simulink model "CCMVgaMotor"  (R2022b) (continued)
== system CCMVgaMotor == (continued)
  VentnActr12TrqSts_VentnActr12TrqSts : Inport  Port=351  OutDataTypeStr=Enum: VentnMotUrgcUnlck  PortDimensions=1
  VentnActr12UrgcExist_VentnActr12UrgcExist : Inport  Port=352  OutDataTypeStr=Enum: VentnActr01UrgcExist  PortDimensions=1
  VentnActr12UrgcPson_VentnActr12UrgcPson : Inport  Port=353  OutDataTypeStr=Enum: VentnMotUrgcPson  PortDimensions=1
  VentnActr12UrgcSts_VentnActr12UrgcSts : Inport  Port=354  OutDataTypeStr=Enum: VentnMotUrgcUnlck  PortDimensions=1
  VentnActr12VoltErr_VentnActr12VoltErr : Inport  Port=355  OutDataTypeStr=Enum: VentnActr01VoltErr  PortDimensions=1
  VentnActr13ActPson_VentnActr13ActPson : Inport  Port=356  OutDataTypeStr=UInt16  PortDimensions=1
  VentnActr13ActPson_VentnActr13ActPson_IsUpdated : Inport  Port=357  OutDataTypeStr=boolean  PortDimensions=1
  VentnActr13BlckInd_VentnActr13BlckInd : Inport  Port=358  OutDataTypeStr=Enum: VentnActr01BlckInd  PortDimensions=1
  VentnActr13BlckSts_VentnActr13BlckSts : Inport  Port=359  OutDataTypeStr=Enum: VentnActr01BlckSts  PortDimensions=1
  VentnActr13BusAdr_VentnActr13BusAdr : Inport  Port=360  OutDataTypeStr=UInt8  PortDimensions=1
  VentnActr13CoilSts_VentnActr13CoilSts : Inport  Port=361  OutDataTypeStr=Enum: VentnMotUrgcUnlck  PortDimensions=1
  VentnActr13DrvDir_VentnActr13DrvDir : Inport  Port=362  OutDataTypeStr=Enum: VentnActr01DrvDir  PortDimensions=1
  VentnActr13ElecErr_VentnActr13ElecErr : Inport  Port=363  OutDataTypeStr=Enum: VentnActr01ElecErr  PortDimensions=1
  VentnActr13Mode_VentnActr13Mode : Inport  Port=364  OutDataTypeStr=Enum: VentnMotMode  PortDimensions=1
  VentnActr13MovDir_VentnActr13MovDir : Inport  Port=365  OutDataTypeStr=Enum: VentnMotDir  PortDimensions=1
  VentnActr13OvrTempErr_VentnActr13OvrTempErr : Inport  Port=366  OutDataTypeStr=Enum: VentnActr01OvrTempErr  PortDimensions=1
  VentnActr13PsonSts_VentnActr13PsonSts : Inport  Port=367  OutDataTypeStr=Enum: VentnMotPsonAvl  PortDimensions=1
  VentnActr13ResetSts_VentnActr13ResetSts : Inport  Port=368  OutDataTypeStr=Enum: VentnActr01ResetSts  PortDimensions=1
  VentnActr13SpclFct_VentnActr13SpclFct : Inport  Port=369  OutDataTypeStr=Enum: VentnActr01SpclFct  PortDimensions=1
  VentnActr13SpdSts_VentnActr13SpdSts : Inport  Port=370  OutDataTypeStr=Enum: VentnActr01SpdSts  PortDimensions=1
  VentnActr13TrqSts_VentnActr13TrqSts : Inport  Port=371  OutDataTypeStr=Enum: VentnMotUrgcUnlck  PortDimensions=1
  VentnActr13UrgcExist_VentnActr13UrgcExist : Inport  Port=372  OutDataTypeStr=Enum: VentnActr01UrgcExist  PortDimensions=1
  VentnActr13UrgcPson_VentnActr13UrgcPson : Inport  Port=373  OutDataTypeStr=Enum: VentnMotUrgcPson  PortDimensions=1
  VentnActr13UrgcSts_VentnActr13UrgcSts : Inport  Port=374  OutDataTypeStr=Enum: VentnMotUrgcUnlck  PortDimensions=1
  VentnActr13VoltErr_VentnActr13VoltErr : Inport  Port=375  OutDataTypeStr=Enum: VentnActr01VoltErr  PortDimensions=1
  VentnActr14ActPson_VentnActr14ActPson : Inport  Port=376  OutDataTypeStr=UInt16  PortDimensions=1
  VentnActr14ActPson_VentnActr14ActPson_IsUpdated : Inport  Port=377  OutDataTypeStr=boolean  PortDimensions=1
  VentnActr14BlckInd_VentnActr14BlckInd : Inport  Port=378  OutDataTypeStr=Enum: VentnActr01BlckInd  PortDimensions=1
  VentnActr14BlckSts_VentnActr14BlckSts : Inport  Port=379  OutDataTypeStr=Enum: VentnActr01BlckSts  PortDimensions=1
  VentnActr14BusAdr_VentnActr14BusAdr : Inport  Port=380  OutDataTypeStr=UInt8  PortDimensions=1
  VentnActr14CoilSts_VentnActr14CoilSts : Inport  Port=381  OutDataTypeStr=Enum: VentnMotUrgcUnlck  PortDimensions=1
  VentnActr14DrvDir_VentnActr14DrvDir : Inport  Port=382  OutDataTypeStr=Enum: VentnActr01DrvDir  PortDimensions=1
  VentnActr14ElecErr_VentnActr14ElecErr : Inport  Port=383  OutDataTypeStr=Enum: VentnActr01ElecErr  PortDimensions=1
  VentnActr14Mode_VentnActr14Mode : Inport  Port=384  OutDataTypeStr=Enum: VentnMotMode  PortDimensions=1
  VentnActr14MovDir_VentnActr14MovDir : Inport  Port=385  OutDataTypeStr=Enum: VentnMotDir  PortDimensions=1
  VentnActr14OvrTempErr_VentnActr14OvrTempErr : Inport  Port=386  OutDataTypeStr=Enum: VentnActr01OvrTempErr  PortDimensions=1
  VentnActr14PsonSts_VentnActr14PsonSts : Inport  Port=387  OutDataTypeStr=Enum: VentnMotPsonAvl  PortDimensions=1
  VentnActr14ResetSts_VentnActr14ResetSts : Inport  Port=388  OutDataTypeStr=Enum: VentnActr01ResetSts  PortDimensions=1
  VentnActr14SpclFct_VentnActr14SpclFct : Inport  Port=389  OutDataTypeStr=Enum: VentnActr01SpclFct  PortDimensions=1
  VentnActr14SpdSts_VentnActr14SpdSts : Inport  Port=390  OutDataTypeStr=Enum: VentnActr01SpdSts  PortDimensions=1
  VentnActr14TrqSts_VentnActr14TrqSts : Inport  Port=391  OutDataTypeStr=Enum: VentnMotUrgcUnlck  PortDimensions=1
  VentnActr14UrgcExist_VentnActr14UrgcExist : Inport  Port=392  OutDataTypeStr=Enum: VentnActr01UrgcExist  PortDimensions=1
  VentnActr14UrgcPson_VentnActr14UrgcPson : Inport  Port=393  OutDataTypeStr=Enum: VentnMotUrgcPson  PortDimensions=1
  VentnActr14UrgcSts_VentnActr14UrgcSts : Inport  Port=394  OutDataTypeStr=Enum: VentnMotUrgcUnlck  PortDimensions=1
  VentnActr14VoltErr_VentnActr14VoltErr : Inport  Port=395  OutDataTypeStr=Enum: VentnActr01VoltErr  PortDimensions=1
  VentnActr15ActPson_VentnActr15ActPson : Inport  Port=396  OutDataTypeStr=UInt16  PortDimensions=1
  VentnActr15ActPson_VentnActr15ActPson_IsUpdated : Inport  Port=397  OutDataTypeStr=boolean  PortDimensions=1
  VentnActr15BlckInd_VentnActr15BlckInd : Inport  Port=398  OutDataTypeStr=Enum: VentnActr01BlckInd  PortDimensions=1
  VentnActr15BlckSts_VentnActr15BlckSts : Inport  Port=399  OutDataTypeStr=Enum: VentnActr01BlckSts  PortDimensions=1
  VentnActr15BusAdr_VentnActr15BusAdr : Inport  Port=400  OutDataTypeStr=UInt8  PortDimensions=1
  VentnActr15CoilSts_VentnActr15CoilSts : Inport  Port=401  OutDataTypeStr=Enum: VentnMotUrgcUnlck  PortDimensions=1
  VentnActr15DrvDir_VentnActr15DrvDir : Inport  Port=402  OutDataTypeStr=Enum: VentnActr01DrvDir  PortDimensions=1
  VentnActr15ElecErr_VentnActr15ElecErr : Inport  Port=403  OutDataTypeStr=Enum: VentnActr01ElecErr  PortDimensions=1
  VentnActr15Mode_VentnActr15Mode : Inport  Port=404  OutDataTypeStr=Enum: VentnMotMode  PortDimensions=1
  VentnActr15MovDir_VentnActr15MovDir : Inport  Port=405  OutDataTypeStr=Enum: VentnMotDir  PortDimensions=1
  VentnActr15OvrTempErr_VentnActr15OvrTempErr : Inport  Port=406  OutDataTypeStr=Enum: VentnActr01OvrTempErr  PortDimensions=1
  VentnActr15PsonSts_VentnActr15PsonSts : Inport  Port=407  OutDataTypeStr=Enum: VentnMotPsonAvl  PortDimensions=1
  VentnActr15ResetSts_VentnActr15ResetSts : Inport  Port=408  OutDataTypeStr=Enum: VentnActr01ResetSts  PortDimensions=1
  VentnActr15SpclFct_VentnActr15SpclFct : Inport  Port=409  OutDataTypeStr=Enum: VentnActr01SpclFct  PortDimensions=1
  VentnActr15SpdSts_VentnActr15SpdSts : Inport  Port=410  OutDataTypeStr=Enum: VentnActr01SpdSts  PortDimensions=1
  VentnActr15TrqSts_VentnActr15TrqSts : Inport  Port=411  OutDataTypeStr=Enum: VentnMotUrgcUnlck  PortDimensions=1
  VentnActr15UrgcExist_VentnActr15UrgcExist : Inport  Port=412  OutDataTypeStr=Enum: VentnActr01UrgcExist  PortDimensions=1
  VentnActr15UrgcPson_VentnActr15UrgcPson : Inport  Port=413  OutDataTypeStr=Enum: VentnMotUrgcPson  PortDimensions=1
  VentnActr15UrgcSts_VentnActr15UrgcSts : Inport  Port=414  OutDataTypeStr=Enum: VentnMotUrgcUnlck  PortDimensions=1
  VentnActr15VoltErr_VentnActr15VoltErr : Inport  Port=415  OutDataTypeStr=Enum: VentnActr01VoltErr  PortDimensions=1
  VentnActr16ActPson_VentnActr16ActPson : Inport  Port=416  OutDataTypeStr=UInt16  PortDimensions=1
  VentnActr16BlckInd_VentnActr16BlckInd : Inport  Port=417  OutDataTypeStr=Enum: VentnActr01BlckInd  PortDimensions=1
  VentnActr16BlckSts_VentnActr16BlckSts : Inport  Port=418  OutDataTypeStr=Enum: VentnActr01BlckSts  PortDimensions=1
  VentnActr16BusAdr_VentnActr16BusAdr : Inport  Port=419  OutDataTypeStr=UInt8  PortDimensions=1
  VentnActr16CoilSts_VentnActr16CoilSts : Inport  Port=420  OutDataTypeStr=Enum: VentnMotUrgcUnlck  PortDimensions=1
  VentnActr16DrvDir_VentnActr16DrvDir : Inport  Port=421  OutDataTypeStr=Enum: VentnActr01DrvDir  PortDimensions=1
  VentnActr16ElecErr_VentnActr16ElecErr : Inport  Port=422  OutDataTypeStr=Enum: VentnActr01ElecErr  PortDimensions=1
  VentnActr16Mode_VentnActr16Mode : Inport  Port=423  OutDataTypeStr=Enum: VentnMotMode  PortDimensions=1
  VentnActr16MovDir_VentnActr16MovDir : Inport  Port=424  OutDataTypeStr=Enum: VentnMotDir  PortDimensions=1
  VentnActr16OvrTempErr_VentnActr16OvrTempErr : Inport  Port=425  OutDataTypeStr=Enum: VentnActr01OvrTempErr  PortDimensions=1
  VentnActr16PsonSts_VentnActr16PsonSts : Inport  Port=426  OutDataTypeStr=Enum: VentnMotPsonAvl  PortDimensions=1
  VentnActr16ResetSts_VentnActr16ResetSts : Inport  Port=427  OutDataTypeStr=Enum: VentnActr01ResetSts  PortDimensions=1
  VentnActr16SpclFct_VentnActr16SpclFct : Inport  Port=428  OutDataTypeStr=Enum: VentnActr01SpclFct  PortDimensions=1
  VentnActr16SpdSts_VentnActr16SpdSts : Inport  Port=429  OutDataTypeStr=Enum: VentnActr01SpdSts  PortDimensions=1
  VentnActr16TrqSts_VentnActr16TrqSts : Inport  Port=430  OutDataTypeStr=Enum: VentnMotUrgcUnlck  PortDimensions=1
  VentnActr16UrgcExist_VentnActr16UrgcExist : Inport  Port=431  OutDataTypeStr=Enum: VentnActr01UrgcExist  PortDimensions=1
  VentnActr16UrgcPson_VentnActr16UrgcPson : Inport  Port=432  OutDataTypeStr=Enum: VentnMotUrgcPson  PortDimensions=1
  VentnActr16UrgcSts_VentnActr16UrgcSts : Inport  Port=433  OutDataTypeStr=Enum: VentnMotUrgcUnlck  PortDimensions=1
  VentnActr16VoltErr_VentnActr16VoltErr : Inport  Port=434  OutDataTypeStr=Enum: VentnActr01VoltErr  PortDimensions=1
  VentnActr17ActPson_VentnActr17ActPson : Inport  Port=435  OutDataTypeStr=UInt16  PortDimensions=1
  VentnActr17ActPson_VentnActr17ActPson_IsUpdated : Inport  Port=436  OutDataTypeStr=boolean  PortDimensions=1
  VentnActr17BlckInd_VentnActr17BlckInd : Inport  Port=437  OutDataTypeStr=Enum: VentnActr01BlckInd  PortDimensions=1
  VentnActr17BlckSts_VentnActr17BlckSts : Inport  Port=438  OutDataTypeStr=Enum: VentnActr01BlckSts  PortDimensions=1
  VentnActr17BusAdr_VentnActr17BusAdr : Inport  Port=439  OutDataTypeStr=UInt8  PortDimensions=1
  VentnActr17CoilSts_VentnActr17CoilSts : Inport  Port=440  OutDataTypeStr=Enum: VentnMotUrgcUnlck  PortDimensions=1
  VentnActr17DrvDir_VentnActr17DrvDir : Inport  Port=441  OutDataTypeStr=Enum: VentnActr01DrvDir  PortDimensions=1
  VentnActr17ElecErr_VentnActr17ElecErr : Inport  Port=442  OutDataTypeStr=Enum: VentnActr01ElecErr  PortDimensions=1
  VentnActr17Mode_VentnActr17Mode : Inport  Port=443  OutDataTypeStr=Enum: VentnMotMode  PortDimensions=1
  VentnActr17MovDir_VentnActr17MovDir : Inport  Port=444  OutDataTypeStr=Enum: VentnMotDir  PortDimensions=1
  VentnActr17OvrTempErr_VentnActr17OvrTempErr : Inport  Port=445  OutDataTypeStr=Enum: VentnActr01OvrTempErr  PortDimensions=1
  VentnActr17PsonSts_VentnActr17PsonSts : Inport  Port=446  OutDataTypeStr=Enum: VentnMotPsonAvl  PortDimensions=1
  VentnActr17ResetSts_VentnActr17ResetSts : Inport  Port=447  OutDataTypeStr=Enum: VentnActr01ResetSts  PortDimensions=1
  VentnActr17SpclFct_VentnActr17SpclFct : Inport  Port=448  OutDataTypeStr=Enum: VentnActr01SpclFct  PortDimensions=1
  VentnActr17SpdSts_VentnActr17SpdSts : Inport  Port=449  OutDataTypeStr=Enum: VentnActr01SpdSts  PortDimensions=1
  VentnActr17TrqSts_VentnActr17TrqSts : Inport  Port=450  OutDataTypeStr=Enum: VentnMotUrgcUnlck  PortDimensions=1
  VentnActr17UrgcExist_VentnActr17UrgcExist : Inport  Port=451  OutDataTypeStr=Enum: VentnActr01UrgcExist  PortDimensions=1
  VentnActr17UrgcPson_VentnActr17UrgcPson : Inport  Port=452  OutDataTypeStr=Enum: VentnMotUrgcPson  PortDimensions=1
  VentnActr17UrgcSts_VentnActr17UrgcSts : Inport  Port=453  OutDataTypeStr=Enum: VentnMotUrgcUnlck  PortDimensions=1
  VentnActr17VoltErr_VentnActr17VoltErr : Inport  Port=454  OutDataTypeStr=Enum: VentnActr01VoltErr  PortDimensions=1
  VentnActr18ActPson_VentnActr18ActPson : Inport  Port=455  OutDataTypeStr=UInt16  PortDimensions=1
  VentnActr18BlckInd_VentnActr18BlckInd : Inport  Port=456  OutDataTypeStr=Enum: VentnActr01BlckInd  PortDimensions=1
  VentnActr18BlckSts_VentnActr18BlckSts : Inport  Port=457  OutDataTypeStr=Enum: VentnActr01BlckInd  PortDimensions=1
  VentnActr18BusAdr_VentnActr18BusAdr : Inport  Port=458  OutDataTypeStr=UInt8  PortDimensions=1
  VentnActr18CoilSts_VentnActr18CoilSts : Inport  Port=459  OutDataTypeStr=Enum: VentnMotUrgcUnlck  PortDimensions=1
  VentnActr18DrvDir_VentnActr18DrvDir : Inport  Port=460  OutDataTypeStr=Enum: VentnActr01DrvDir  PortDimensions=1
  VentnActr18ElecErr_VentnActr18ElecErr : Inport  Port=461  OutDataTypeStr=Enum: VentnActr01ElecErr  PortDimensions=1
  VentnActr18Mode_VentnActr18Mode : Inport  Port=462  OutDataTypeStr=Enum: VentnMotMode  PortDimensions=1
  VentnActr18MovDir_VentnActr18MovDir : Inport  Port=463  OutDataTypeStr=Enum: VentnMotDir  PortDimensions=1
  VentnActr18OvrTempErr_VentnActr18OvrTempErr : Inport  Port=464  OutDataTypeStr=Enum: VentnActr01OvrTempErr  PortDimensions=1
  VentnActr18PsonSts_VentnActr18PsonSts : Inport  Port=465  OutDataTypeStr=Enum: VentnMotPsonAvl  PortDimensions=1
  VentnActr18ResetSts_VentnActr18ResetSts : Inport  Port=466  OutDataTypeStr=Enum: VentnActr01ResetSts  PortDimensions=1
  VentnActr18SpclFct_VentnActr18SpclFct : Inport  Port=467  OutDataTypeStr=Enum: VentnActr01SpclFct  PortDimensions=1
  VentnActr18SpdSts_VentnActr18SpdSts : Inport  Port=468  OutDataTypeStr=Enum: VentnActr01SpdSts  PortDimensions=1
  VentnActr18TrqSts_VentnActr18TrqSts : Inport  Port=469  OutDataTypeStr=Enum: VentnMotUrgcUnlck  PortDimensions=1
  VentnActr18UrgcExist_VentnActr18UrgcExist : Inport  Port=470  OutDataTypeStr=Enum: VentnActr01UrgcExist  PortDimensions=1
  VentnActr18UrgcPson_VentnActr18UrgcPson : Inport  Port=471  OutDataTypeStr=Enum: VentnMotUrgcPson  PortDimensions=1
  VentnActr18UrgcSts_VentnActr18UrgcSts : Inport  Port=472  OutDataTypeStr=Enum: VentnMotUrgcUnlck  PortDimensions=1
  VentnActr18VoltErr_VentnActr18VoltErr : Inport  Port=473  OutDataTypeStr=Enum: VentnActr01ElecErr  PortDimensions=1
  VentnActr19ActPson_VentnActr19ActPson : Inport  Port=474  OutDataTypeStr=UInt16  PortDimensions=1
  VentnActr19ActPson_VentnActr19ActPson_IsUpdated : Inport  Port=475  OutDataTypeStr=boolean  PortDimensions=1
  VentnActr19BlckInd_VentnActr19BlckInd : Inport  Port=476  OutDataTypeStr=Enum: VentnActr01BlckInd  PortDimensions=1
  VentnActr19BlckSts_VentnActr19BlckSts : Inport  Port=477  OutDataTypeStr=Enum: VentnActr01BlckInd  PortDimensions=1
  VentnActr19BusAdr_VentnActr19BusAdr : Inport  Port=478  OutDataTypeStr=UInt8  PortDimensions=1
  VentnActr19CoilSts_VentnActr19CoilSts : Inport  Port=479  OutDataTypeStr=Enum: VentnMotUrgcUnlck  PortDimensions=1
  VentnActr19DrvDir_VentnActr19DrvDir : Inport  Port=480  OutDataTypeStr=Enum: VentnActr01DrvDir  PortDimensions=1
  VentnActr19ElecErr_VentnActr19ElecErr : Inport  Port=481  OutDataTypeStr=Enum: VentnActr01ElecErr  PortDimensions=1
  VentnActr19Mode_VentnActr19Mode : Inport  Port=482  OutDataTypeStr=Enum: VentnMotMode  PortDimensions=1
  VentnActr19MovDir_VentnActr19MovDir : Inport  Port=483  OutDataTypeStr=Enum: VentnMotDir  PortDimensions=1
  VentnActr19OvrTempErr_VentnActr19OvrTempErr : Inport  Port=484  OutDataTypeStr=Enum: VentnActr01OvrTempErr  PortDimensions=1
  VentnActr19PsonSts_VentnActr19PsonSts : Inport  Port=485  OutDataTypeStr=Enum: VentnMotPsonAvl  PortDimensions=1
  VentnActr19ResetSts_VentnActr19ResetSts : Inport  Port=486  OutDataTypeStr=Enum: VentnActr01ResetSts  PortDimensions=1
  VentnActr19SpclFct_VentnActr19SpclFct : Inport  Port=487  OutDataTypeStr=Enum: VentnActr01SpclFct  PortDimensions=1
  VentnActr19SpdSts_VentnActr19SpdSts : Inport  Port=488  OutDataTypeStr=Enum: VentnActr01SpdSts  PortDimensions=1
  VentnActr19TrqSts_VentnActr19TrqSts : Inport  Port=489  OutDataTypeStr=Enum: VentnMotUrgcUnlck  PortDimensions=1
  VentnActr19UrgcExist_VentnActr19UrgcExist : Inport  Port=490  OutDataTypeStr=Enum: VentnActr01UrgcExist  PortDimensions=1
  VentnActr19UrgcPson_VentnActr19UrgcPson : Inport  Port=491  OutDataTypeStr=Enum: VentnMotUrgcPson  PortDimensions=1
  VentnActr19UrgcSts_VentnActr19UrgcSts : Inport  Port=492  OutDataTypeStr=Enum: VentnMotUrgcUnlck  PortDimensions=1
  VentnActr19VoltErr_VentnActr19VoltErr : Inport  Port=493  OutDataTypeStr=Enum: VentnActr01VoltErr  PortDimensions=1
  VentnActr20ActPson_VentnActr20ActPson : Inport  Port=494  OutDataTypeStr=UInt16  PortDimensions=1
  VentnActr20ActPson_VentnActr20ActPson_IsUpdated : Inport  Port=495  OutDataTypeStr=boolean  PortDimensions=1
  VentnActr20BlckInd_VentnActr20BlckInd : Inport  Port=496  OutDataTypeStr=Enum: VentnActr01BlckInd  PortDimensions=1
  VentnActr20BlckSts_VentnActr20BlckSts : Inport  Port=497  OutDataTypeStr=Enum: VentnActr01BlckSts  PortDimensions=1
  VentnActr20BusAdr_VentnActr20BusAdr : Inport  Port=498  OutDataTypeStr=UInt8  PortDimensions=1
  VentnActr20CoilSts_VentnActr20CoilSts : Inport  Port=499  OutDataTypeStr=Enum: VentnMotUrgcUnlck  PortDimensions=1
  VentnActr20DrvDir_VentnActr20DrvDir : Inport  Port=500  OutDataTypeStr=Enum: VentnActr01DrvDir  PortDimensions=1
  VentnActr20ElecErr_VentnActr20ElecErr : Inport  Port=501  OutDataTypeStr=Enum: VentnActr01ElecErr  PortDimensions=1
  VentnActr20Mode_VentnActr20Mode : Inport  Port=502  OutDataTypeStr=Enum: VentnMotMode  PortDimensions=1
  VentnActr20MovDir_VentnActr20MovDir : Inport  Port=503  OutDataTypeStr=Enum: VentnMotDir  PortDimensions=1
  VentnActr20OvrTempErr_VentnActr20OvrTempErr : Inport  Port=504  OutDataTypeStr=Enum: VentnActr01OvrTempErr  PortDimensions=1
  VentnActr20PsonSts_VentnActr20PsonSts : Inport  Port=505  OutDataTypeStr=Enum: VentnMotPsonAvl  PortDimensions=1
  VentnActr20ResetSts_VentnActr20ResetSts : Inport  Port=506  OutDataTypeStr=Enum: VentnActr01ResetSts  PortDimensions=1
  VentnActr20SpclFct_VentnActr20SpclFct : Inport  Port=507  OutDataTypeStr=Enum: VentnActr01SpclFct  PortDimensions=1
  VentnActr20SpdSts_VentnActr20SpdSts : Inport  Port=508  OutDataTypeStr=Enum: VentnActr01SpdSts  PortDimensions=1
  VentnActr20TrqSts_VentnActr20TrqSts : Inport  Port=509  OutDataTypeStr=Enum: VentnMotUrgcUnlck  PortDimensions=1
  VentnActr20UrgcExist_VentnActr20UrgcExist : Inport  Port=510  OutDataTypeStr=Enum: VentnActr01UrgcExist  PortDimensions=1
  VentnActr20UrgcPson_VentnActr20UrgcPson : Inport  Port=511  OutDataTypeStr=Enum: VentnMotUrgcPson  PortDimensions=1
  VentnActr20UrgcSts_VentnActr20UrgcSts : Inport  Port=512  OutDataTypeStr=Enum: VentnMotUrgcUnlck  PortDimensions=1
  VentnActr20VoltErr_VentnActr20VoltErr : Inport  Port=513  OutDataTypeStr=Enum: VentnActr01VoltErr  PortDimensions=1
  VGAStopDuePowerLimit_VGAStopDuePowerLimit : Inport  Port=514  OutDataTypeStr=Boolean  PortDimensions=1
  BswLifeCycle_LifeCycle : Inport  Port=515  OutDataTypeStr=Enum: CCMLifeCycleModeGroup  PortDimensions=1
  CCMVGAMotor_VentnMotAdr2_sys : Subsystem
  CCMVGAMotor_VentnMotAdr_sys : Subsystem
  CCMVgaMotor_50msRunnable_sys : Subsystem
  CCMVgaMotor_Init : Subsystem
  CCMVgaMotor_Runnable_sys : Subsystem
  NvMBlockVgaMtrCalData : DataStoreMemory  DataStoreName=NvMBlockVgaMtrCalData  InitialValue=NvMBlockVgaMtrCalDataInit  ShowAdditionalParam=off  Dimensions=210  StateMustResolveToSignalObject=on
  VgaCaliServive : Subsystem
  VgaDtcService : Subsystem
  VgaIOCtrlService : Subsystem
  VgaNvmService : Subsystem
  VgaParaService : Subsystem
  VentnMotAdr_VentnMotAdr : Outport  OutDataTypeStr=UInt8  PortDimensions=1
  VentnMotAdr2_VentnMotAdr2 : Outport  Port=2  OutDataTypeStr=UInt8  PortDimensions=1
  VentnMotBlockCmd_VentnMotBlockCmd : Outport  Port=3  OutDataTypeStr=Enum: VentnMotBlockCmd  PortDimensions=1
  VentnMotBlockCmd2_VentnMotBlockCmd2 : Outport  Port=4  OutDataTypeStr=Enum: VentnMotBlockCmd  PortDimensions=1
  VentnMotClearReq_VentnMotClearReq : Outport  Port=5  OutMin=0  OutMax=15  OutDataTypeStr=UInt4  PortDimensions=1
  VentnMotClearReq2_VentnMotClearReq2 : Outport  Port=6  OutMin=0  OutMax=15  OutDataTypeStr=UInt4  PortDimensions=1
  VentnMotCoilPwrOn_VentnMotCoilPwrOn : Outport  Port=7  OutDataTypeStr=Enum: VentnMotUrgcUnlck  PortDimensions=1
  VentnMotCoilPwrOn2_VentnMotCoilPwrOn2 : Outport  Port=8  OutDataTypeStr=Enum: VentnMotUrgcUnlck  PortDimensions=1
  VentnMotDir_VentnMotDir : Outport  Port=9  OutDataTypeStr=Enum: VentnMotDir  PortDimensions=1
  VentnMotDir2_VentnMotDir2 : Outport  Port=10  OutDataTypeStr=Enum: VentnMotDir  PortDimensions=1
  VentnMotMode_VentnMotMode : Outport  Port=11  OutDataTypeStr=Enum: VentnMotMode  PortDimensions=1
  VentnMotMode2_VentnMotMode2 : Outport  Port=12  OutDataTypeStr=Enum: VentnMotMode  PortDimensions=1
  VentnMotPsonAvl_VentnMotPsonAvl : Outport  Port=13  OutDataTypeStr=Enum: VentnMotPsonAvl  PortDimensions=1
  VentnMotPsonAvl2_VentnMotPsonAvl2 : Outport  Port=14  OutDataTypeStr=Enum: VentnMotPsonAvl  PortDimensions=1
  VentnMotSaveData_VentnMotSaveData : Outport  Port=15  OutDataTypeStr=Enum: VentnMotSaveData  PortDimensions=1
  VentnMotSaveData2_VentnMotSaveData2 : Outport  Port=16  OutDataTypeStr=Enum: VentnMotSaveData  PortDimensions=1
  VentnMotSpdSet_VentnMotSpdSet : Outport  Port=17  OutDataTypeStr=Enum: VentnMotSpdSet  PortDimensions=1
  VentnMotSpdSet2_VentnMotSpdSet2 : Outport  Port=18  OutDataTypeStr=Enum: VentnMotSpdSet  PortDimensions=1
  VentnMotStrtPson_VentnMotStrtPson : Outport  Port=19  OutDataTypeStr=UInt16  PortDimensions=1
  VentnMotStrtPson2_VentnMotStrtPson2 : Outport  Port=20  OutDataTypeStr=UInt16  PortDimensions=1
  VentnMotTargtPson_VentnMotTargtPson : Outport  Port=21  OutDataTypeStr=UInt16  PortDimensions=1
  VentnMotTargtPson2_VentnMotTargtPson2 : Outport  Port=22  OutDataTypeStr=UInt16  PortDimensions=1
  VentnMotUrgcPson_VentnMotUrgcPson : Outport  Port=23  OutDataTypeStr=Enum: VentnMotUrgcPson  PortDimensions=1
  VentnMotUrgcPson2_VentnMotUrgcPson2 : Outport  Port=24  OutDataTypeStr=Enum: VentnMotUrgcPson  PortDimensions=1
  VentnMotUrgcUnlck_VentnMotUrgcUnlck : Outport  Port=25  OutDataTypeStr=Enum: VentnMotUrgcUnlck  PortDimensions=1
  VentnMotUrgcUnlck2_VentnMotUrgcUnlck2 : Outport  Port=26  OutDataTypeStr=Enum: VentnMotUrgcUnlck  PortDimensions=1
  wires:
  CarCfgForClimaCtrl_CarCfgForAirClng/1 -> CCMVgaMotor_Runnable_sys/1(CarCfgForClimaCtrl_CarCfgForAirClng_read)
  CarCfgForClimaCtrl_CarCfgForAirClnrAdv/1 -> CCMVgaMotor_Runnable_sys/2(CarCfgForClimaCtrl_CarCfgForAirClnrAdv_read)
  CarCfgForClimaCtrl_CarCfgForAirFrag/1 -> CCMVgaMotor_Runnable_sys/3(CarCfgForClimaCtrl_CarCfgForAirFrag_read)
  CarCfgForClimaCtrl_CarCfgForAirMtrlSnsr/1 -> CCMVgaMotor_Runnable_sys/4(CarCfgForClimaCtrl_CarCfgForAirMtrlSnsr_read)
  CarCfgForClimaCtrl_CarCfgForAirQly/1 -> CCMVgaMotor_Runnable_sys/5(CarCfgForClimaCtrl_CarCfgForAirQly_read)
  CarCfgForClimaCtrl_CarCfgForAmntOfSeat/1 -> CCMVgaMotor_Runnable_sys/6(CarCfgForClimaCtrl_CarCfgForAmntOfSeat_read)
  CarCfgForClimaCtrl_CarCfgForAuxWtrPmp/1 -> CCMVgaMotor_Runnable_sys/7(CarCfgForClimaCtrl_CarCfgForAuxWtrPmp_read)
  CarCfgForClimaCtrl_CarCfgForBypVlvInHvac/1 -> CCMVgaMotor_Runnable_sys/8(CarCfgForClimaCtrl_CarCfgForBypVlvInHvac_read)
  CarCfgForClimaCtrl_CarCfgForClsVlvInHvac/1 -> CCMVgaMotor_Runnable_sys/9(CarCfgForClimaCtrl_CarCfgForClsVlvInHvac_read)
  CarCfgForClimaCtrl_CarCfgForCmprAcTyp/1 -> CCMVgaMotor_Runnable_sys/10(CarCfgForClimaCtrl_CarCfgForCmprAcTyp_read)
  CarCfgForClimaCtrl_CarCfgForCmptmtClng/1 -> CCMVgaMotor_Runnable_sys/11(CarCfgForClimaCtrl_CarCfgForCmptmtClng_read)
  CarCfgForClimaCtrl_CarCfgForElecVentAdjmt/1 -> CCMVgaMotor_Runnable_sys/12(CarCfgForClimaCtrl_CarCfgForElecVentAdjmt_read)
  CarCfgForClimaCtrl_CarCfgForFlcTyp/1 -> CCMVgaMotor_Runnable_sys/13(CarCfgForClimaCtrl_CarCfgForFlcTyp_read)
  CarCfgForClimaCtrl_CarCfgForFoldCSD/1 -> CCMVgaMotor_Runnable_sys/14(CarCfgForClimaCtrl_CarCfgForFoldCSD_read)
  CarCfgForClimaCtrl_CarCfgForFuTyp/1 -> CCMVgaMotor_Runnable_sys/15(CarCfgForClimaCtrl_CarCfgForFuTyp_read)
  CarCfgForClimaCtrl_CarCfgForGlassTyp/1 -> CCMVgaMotor_Runnable_sys/16(CarCfgForClimaCtrl_CarCfgForGlassTyp_read)
  CarCfgForClimaCtrl_CarCfgForHeatPmp/1 -> CCMVgaMotor_Runnable_sys/17(CarCfgForClimaCtrl_CarCfgForHeatPmp_read)
  CarCfgForClimaCtrl_CarCfgForHeatrDurgDrvg/1 -> CCMVgaMotor_Runnable_sys/18(CarCfgForClimaCtrl_CarCfgForHeatrDurgDrvg_read)
  CarCfgForClimaCtrl_CarCfgForHeatrTyp/1 -> CCMVgaMotor_Runnable_sys/19(CarCfgForClimaCtrl_CarCfgForHeatrTyp_read)
  CarCfgForClimaCtrl_CarCfgForHvacFilTyp/1 -> CCMVgaMotor_Runnable_sys/20(CarCfgForClimaCtrl_CarCfgForHvacFilTyp_read)
  CarCfgForClimaCtrl_CarCfgForHvacFlrSnsrForT/1 -> CCMVgaMotor_Runnable_sys/21(CarCfgForClimaCtrl_CarCfgForHvacFlrSnsrForT_read)
  CarCfgForClimaCtrl_CarCfgForHvacHeatrSnsrT/1 -> CCMVgaMotor_Runnable_sys/22(CarCfgForClimaCtrl_CarCfgForHvacHeatrSnsrT_read)
  CarCfgForClimaCtrl_CarCfgForHvacRe/1 -> CCMVgaMotor_Runnable_sys/23(CarCfgForClimaCtrl_CarCfgForHvacRe_read)
  CarCfgForClimaCtrl_CarCfgForHvacTyp/1 -> CCMVgaMotor_Runnable_sys/24(CarCfgForClimaCtrl_CarCfgForHvacTyp_read)
  CarCfgForClimaCtrl_CarCfgForLoVoltElecCfg/1 -> CCMVgaMotor_Runnable_sys/25(CarCfgForClimaCtrl_CarCfgForLoVoltElecCfg_read)
  CarCfgForClimaCtrl_CarCfgForNavSysandIntnmap/1 -> CCMVgaMotor_Runnable_sys/26(CarCfgForClimaCtrl_CarCfgForNavSysandIntnmap_read)
  CarCfgForClimaCtrl_CarCfgForNrOfDoor/1 -> CCMVgaMotor_Runnable_sys/27(CarCfgForClimaCtrl_CarCfgForNrOfDoor_read)
  CarCfgForClimaCtrl_CarCfgForPipeInFuTank/1 -> CCMVgaMotor_Runnable_sys/28(CarCfgForClimaCtrl_CarCfgForPipeInFuTank_read)
  CarCfgForClimaCtrl_CarCfgForPreClng/1 -> CCMVgaMotor_Runnable_sys/29(CarCfgForClimaCtrl_CarCfgForPreClng_read)
  CarCfgForClimaCtrl_CarCfgForPrkgCDSNotAvl/1 -> CCMVgaMotor_Runnable_sys/30(CarCfgForClimaCtrl_CarCfgForPrkgCDSNotAvl_read)
  CarCfgForClimaCtrl_CarCfgForPrkgClima/1 -> CCMVgaMotor_Runnable_sys/31(CarCfgForClimaCtrl_CarCfgForPrkgClima_read)
  CarCfgForClimaCtrl_CarCfgForPrpsnTyp/1 -> CCMVgaMotor_Runnable_sys/32(CarCfgForClimaCtrl_CarCfgForPrpsnTyp_read)
  CarCfgForClimaCtrl_CarCfgForRemEngStrtPreCdn/1 -> CCMVgaMotor_Runnable_sys/33(CarCfgForClimaCtrl_CarCfgForRemEngStrtPreCdn_read)
  CarCfgForClimaCtrl_CarCfgForRoofHatch/1 -> CCMVgaMotor_Runnable_sys/34(CarCfgForClimaCtrl_CarCfgForRoofHatch_read)
  CarCfgForClimaCtrl_CarCfgForSeatHeatgCal/1 -> CCMVgaMotor_Runnable_sys/35(CarCfgForClimaCtrl_CarCfgForSeatHeatgCal_read)
  CarCfgForClimaCtrl_CarCfgForSeatHeatgForRowFirst/1 -> CCMVgaMotor_Runnable_sys/36(CarCfgForClimaCtrl_CarCfgForSeatHeatgForRowFirst_read)
  CarCfgForClimaCtrl_CarCfgForSeatHeatgForRowSec/1 -> CCMVgaMotor_Runnable_sys/37(CarCfgForClimaCtrl_CarCfgForSeatHeatgForRowSec_read)
  CarCfgForClimaCtrl_CarCfgForSeatVentnForRowFirst/1 -> CCMVgaMotor_Runnable_sys/38(CarCfgForClimaCtrl_CarCfgForSeatVentnForRowFirst_read)
  CarCfgForClimaCtrl_CarCfgForSeatVentnForRowSec/1 -> CCMVgaMotor_Runnable_sys/39(CarCfgForClimaCtrl_CarCfgForSeatVentnForRowSec_read)
  CarCfgForClimaCtrl_CarCfgForSecRowVentnCenter/1 -> CCMVgaMotor_Runnable_sys/40(CarCfgForClimaCtrl_CarCfgForSecRowVentnCenter_read)
  CarCfgForClimaCtrl_CarCfgForSecRowVentnSide/1 -> CCMVgaMotor_Runnable_sys/41(CarCfgForClimaCtrl_CarCfgForSecRowVentnSide_read)
  CarCfgForClimaCtrl_CarCfgForSmallFlowCyc/1 -> CCMVgaMotor_Runnable_sys/42(CarCfgForClimaCtrl_CarCfgForSmallFlowCyc_read)
  CarCfgForClimaCtrl_CarCfgForSmkSnsr/1 -> CCMVgaMotor_Runnable_sys/43(CarCfgForClimaCtrl_CarCfgForSmkSnsr_read)
  CarCfgForClimaCtrl_CarCfgForSnsrForCmptmtT/1 -> CCMVgaMotor_Runnable_sys/44(CarCfgForClimaCtrl_CarCfgForSnsrForCmptmtT_read)
  CarCfgForClimaCtrl_CarCfgForSnsrHumPrsnt/1 -> CCMVgaMotor_Runnable_sys/45(CarCfgForClimaCtrl_CarCfgForSnsrHumPrsnt_read)
  CarCfgForClimaCtrl_CarCfgForSteerWhlHeatg/1 -> CCMVgaMotor_Runnable_sys/46(CarCfgForClimaCtrl_CarCfgForSteerWhlHeatg_read)
  CarCfgForClimaCtrl_CarCfgForSteerWhlPosn/1 -> CCMVgaMotor_Runnable_sys/47(CarCfgForClimaCtrl_CarCfgForSteerWhlPosn_read)
  CarCfgForClimaCtrl_CarCfgForSunSnsr/1 -> CCMVgaMotor_Runnable_sys/48(CarCfgForClimaCtrl_CarCfgForSunSnsr_read)
  CarCfgForClimaCtrl_CarCfgForTelmNodTyp/1 -> CCMVgaMotor_Runnable_sys/49(CarCfgForClimaCtrl_CarCfgForTelmNodTyp_read)
  CarCfgForClimaCtrl_CarCfgForThermMngt/1 -> CCMVgaMotor_Runnable_sys/50(CarCfgForClimaCtrl_CarCfgForThermMngt_read)
  CarCfgForClimaCtrl_CarCfgForVehTyp/1 -> CCMVgaMotor_Runnable_sys/51(CarCfgForClimaCtrl_CarCfgForVehTyp_read)
  CarCfgForClimaCtrl_CarCfgForVgaMotorExistSts/1 -> CCMVgaMotor_Runnable_sys/52(CarCfgForClimaCtrl_CarCfgForVgaMotorExistSts_read)
  CarCfgForClimaCtrl_CarCfgForWindHeatd/1 -> CCMVgaMotor_Runnable_sys/53(CarCfgForClimaCtrl_CarCfgForWindHeatd_read)
  CarCfgForClimaCtrl_CarCfgForAutDemst/1 -> CCMVgaMotor_Runnable_sys/54(CarCfgForClimaCtrl_CarCfgForAutDemst_read)
  CarCfgForClimaCtrl_CarCfgForExtVentnClimaCtrl/1 -> CCMVgaMotor_Runnable_sys/55(CarCfgForClimaCtrl_CarCfgForExtVentnClimaCtrl_read)
  CarCfgForClimaCtrl_CarCfgForStartDevice/1 -> CCMVgaMotor_Runnable_sys/56(CarCfgForClimaCtrl_CarCfgForStartDevice_read)
  HmiElecAirSwngReq_HmiElecAirSwngReq/1 -> CCMVgaMotor_Runnable_sys/57(HmiElecAirSwngReq_HmiElecAirSwngReq_read)
  LIN2ScheTableReq_Val/1 -> CCMVgaMotor_Runnable_sys/58(LIN2ScheTableReq_Val_read)
  SysServCarCfgSts_SysServCarID/1 -> CCMVgaMotor_Runnable_sys/59(SysServCarCfgSts_SysServCarID_read)
  SysServCarCfgSts_SysServCCPAQSSts/1 -> CCMVgaMotor_Runnable_sys/60(SysServCarCfgSts_SysServCCPAQSSts_read)
  SysServCarCfgSts_SysServCCPHEXSensorSts/1 -> CCMVgaMotor_Runnable_sys/61(SysServCarCfgSts_SysServCCPHEXSensorSts_read)
  SysServCarCfgSts_SysServCCPLin2AFUSts/1 -> CCMVgaMotor_Runnable_sys/62(SysServCarCfgSts_SysServCCPLin2AFUSts_read)
  SysServCarCfgSts_SysServCCPLin2AIUSts/1 -> CCMVgaMotor_Runnable_sys/63(SysServCarCfgSts_SysServCCPLin2AIUSts_read)
  SysServCarCfgSts_SysServCCPLin2CDSSts/1 -> CCMVgaMotor_Runnable_sys/64(SysServCarCfgSts_SysServCCPLin2CDSSts_read)
  SysServCarCfgSts_SysServCCPLin2PMSISts/1 -> CCMVgaMotor_Runnable_sys/65(SysServCarCfgSts_SysServCCPLin2PMSISts_read)
  SysServCarCfgSts_SysServCCPLin3VGASts/1 -> CCMVgaMotor_Runnable_sys/66(SysServCarCfgSts_SysServCCPLin3VGASts_read)
  SysServCarCfgSts_SysServDUAirVentSnrSts/1 -> CCMVgaMotor_Runnable_sys/67(SysServCarCfgSts_SysServDUAirVentSnrSts_read)
  SysServCarCfgSts_SysServDUBlowerPwmSignSts/1 -> CCMVgaMotor_Runnable_sys/68(SysServCarCfgSts_SysServDUBlowerPwmSignSts_read)
  SysServCarCfgSts_SysServDUBlowerPwmSts/1 -> CCMVgaMotor_Runnable_sys/69(SysServCarCfgSts_SysServDUBlowerPwmSts_read)
  SysServCarCfgSts_SysServDUDCDefrostSts/1 -> CCMVgaMotor_Runnable_sys/70(SysServCarCfgSts_SysServDUDCDefrostSts_read)
  SysServCarCfgSts_SysServDUDCModeSts/1 -> CCMVgaMotor_Runnable_sys/71(SysServCarCfgSts_SysServDUDCModeSts_read)
  SysServCarCfgSts_SysServDUDCRecSts/1 -> CCMVgaMotor_Runnable_sys/72(SysServCarCfgSts_SysServDUDCRecSts_read)
  SysServCarCfgSts_SysServDUDCSModeSts/1 -> CCMVgaMotor_Runnable_sys/73(SysServCarCfgSts_SysServDUDCSModeSts_read)
  SysServCarCfgSts_SysServDUDCSTLSts/1 -> CCMVgaMotor_Runnable_sys/74(SysServCarCfgSts_SysServDUDCSTLSts_read)
  SysServCarCfgSts_SysServDUDCTLSts/1 -> CCMVgaMotor_Runnable_sys/75(SysServCarCfgSts_SysServDUDCTLSts_read)
  SysServCarCfgSts_SysServDUDCTRSts/1 -> CCMVgaMotor_Runnable_sys/76(SysServCarCfgSts_SysServDUDCTRSts_read)
  SysServCarCfgSts_SysServDUFLFootSts/1 -> CCMVgaMotor_Runnable_sys/77(SysServCarCfgSts_SysServDUFLFootSts_read)
  SysServCarCfgSts_SysServDUFLVentSts/1 -> CCMVgaMotor_Runnable_sys/78(SysServCarCfgSts_SysServDUFLVentSts_read)
  SysServCarCfgSts_SysServDUFRFootSts/1 -> CCMVgaMotor_Runnable_sys/79(SysServCarCfgSts_SysServDUFRFootSts_read)
  SysServCarCfgSts_SysServDUFRVentSts/1 -> CCMVgaMotor_Runnable_sys/80(SysServCarCfgSts_SysServDUFRVentSts_read)
  SysServCarCfgSts_SysServDUIncarMotorSts/1 -> CCMVgaMotor_Runnable_sys/81(SysServCarCfgSts_SysServDUIncarMotorSts_read)
  SysServCarCfgSts_SysServDULin1HBMFSts/1 -> CCMVgaMotor_Runnable_sys/82(SysServCarCfgSts_SysServDULin1HBMFSts_read)
  SysServCarCfgSts_SysServDULin4HFAFSts/1 -> CCMVgaMotor_Runnable_sys/83(SysServCarCfgSts_SysServDULin4HFAFSts_read)
  SysServCarCfgSts_SysServDUSFLFootSts/1 -> CCMVgaMotor_Runnable_sys/84(SysServCarCfgSts_SysServDUSFLFootSts_read)
  SysServCarCfgSts_SysServDUSFLVentSts/1 -> CCMVgaMotor_Runnable_sys/85(SysServCarCfgSts_SysServDUSFLVentSts_read)
  SysServCarCfgSts_SysServDUSFRFootSts/1 -> CCMVgaMotor_Runnable_sys/86(SysServCarCfgSts_SysServDUSFRFootSts_read)
  SysServCarCfgSts_SysServDUSFRVentSts/1 -> CCMVgaMotor_Runnable_sys/87(SysServCarCfgSts_SysServDUSFRVentSts_read)
  SysServCarCfgSts_SysServDUSnsrForFCmptmtTSts/1 -> CCMVgaMotor_Runnable_sys/88(SysServCarCfgSts_SysServDUSnsrForFCmptmtTSts_read)
  SysServCarCfgSts_SysServDUSnsrForRair/1 -> CCMVgaMotor_Runnable_sys/89(SysServCarCfgSts_SysServDUSnsrForRair_read)
  SysServCarCfgSts_SysServDUSnsrForRCmptmtTSts/1 -> CCMVgaMotor_Runnable_sys/90(SysServCarCfgSts_SysServDUSnsrForRCmptmtTSts_read)
  VehModMngtGlbSafe1_VehModMngtGlbSafe1/1 -> CCMVgaMotor_Runnable_sys/91(VehModMngtGlbSafe1_VehModMngtGlbSafe1_read)
  BatLoadBtrMSts_BatLoadBtrMSts/1 -> CCMVgaMotor_Runnable_sys/92(BatLoadBtrMSts_BatLoadBtrMSts_read)
  BatSysBtrMSts_BatSysBtrMSts/1 -> CCMVgaMotor_Runnable_sys/93(BatSysBtrMSts_BatSysBtrMSts_read)
  VgaMotSetPowerOnInit_PowerOnInit/1 -> CCMVgaMotor_Runnable_sys/94(VgaMotSetPowerOnInit_PowerOnInit_read)
  CarCfgForClimaCtrl_CarCfgForBRAND/1 -> CCMVgaMotor_Runnable_sys/95(CarCfgForClimaCtrl_CarCfgForBRAND_read)
  CarCfgForClimaCtrl_CarCfgForHeadRadar/1 -> CCMVgaMotor_Runnable_sys/96(CarCfgForClimaCtrl_CarCfgForHeadRadar_read)
  CarCfgForClimaCtrl_CarCfgForInteriorViewCamera/1 -> CCMVgaMotor_Runnable_sys/97(CarCfgForClimaCtrl_CarCfgForInteriorViewCamera_read)
  CarCfgForClimaCtrl_CarCfgForLifeDetection/1 -> CCMVgaMotor_Runnable_sys/98(CarCfgForClimaCtrl_CarCfgForLifeDetection_read)
  CarCfgForClimaCtrl_CarCfgForPowerSeats/1 -> CCMVgaMotor_Runnable_sys/99(CarCfgForClimaCtrl_CarCfgForPowerSeats_read)
  CarCfgForClimaCtrl_CarCfgForPTCInFrontHVAC/1 -> CCMVgaMotor_Runnable_sys/100(CarCfgForClimaCtrl_CarCfgForPTCInFrontHVAC_read)
  CarCfgForClimaCtrl_CarCfgForVentLiftMotorExiSts/1 -> CCMVgaMotor_Runnable_sys/101(CarCfgForClimaCtrl_CarCfgForVentLiftMotorExiSts_read)
  CarCfgForClimaCtrl_CarCfgForWadingAssistSystem/1 -> CCMVgaMotor_Runnable_sys/102(CarCfgForClimaCtrl_CarCfgForWadingAssistSystem_read)
  SysServCarCfgSts_SysServCCPBGMNum/1 -> CCMVgaMotor_Runnable_sys/105(SysServCarCfgSts_SysServCCPBGMNum_read)
  SysServCarCfgSts_SysServCCPLin2AQSSts/1 -> CCMVgaMotor_Runnable_sys/106(SysServCarCfgSts_SysServCCPLin2AQSSts_read)
  SysServCarCfgSts_SysServDUBlowerPwmSignTypeSts/1 -> CCMVgaMotor_Runnable_sys/107(SysServCarCfgSts_SysServDUBlowerPwmSignTypeSts_read)
  SysServCarCfgSts_SysServDUDCFLMdeSts/1 -> CCMVgaMotor_Runnable_sys/108(SysServCarCfgSts_SysServDUDCFLMdeSts_read)
  SysServCarCfgSts_SysServDUReBlowerPwmSignSts/1 -> CCMVgaMotor_Runnable_sys/109(SysServCarCfgSts_SysServDUReBlowerPwmSignSts_read)
  SysServCarCfgSts_SysServDUReBlowerPwmSts/1 -> CCMVgaMotor_Runnable_sys/110(SysServCarCfgSts_SysServDUReBlowerPwmSts_read)
  SysServCarCfgSts_SysServLowSideRETXVSts/1 -> CCMVgaMotor_Runnable_sys/111(SysServCarCfgSts_SysServLowSideRETXVSts_read)
  VentnActr01ActPson_VentnActr01ActPson/1 -> CCMVgaMotor_Runnable_sys/112(VentnActr01ActPson_VentnActr01ActPson_read)
  VentnActr01ActPson_VentnActr01ActPson_IsUpdated/1 -> CCMVgaMotor_Runnable_sys/113(VentnActr01ActPson_VentnActr01ActPson_IsUpdated_read)
  VentnActr01BlckInd_VentnActr01BlckInd/1 -> CCMVgaMotor_Runnable_sys/114(VentnActr01BlckInd_VentnActr01BlckInd_read)
  VentnActr01BlckSts_VentnActr01BlckSts/1 -> CCMVgaMotor_Runnable_sys/115(VentnActr01BlckSts_VentnActr01BlckSts_read)
  VentnActr01BusAdr_VentnActr01BusAdr/1 -> CCMVgaMotor_Runnable_sys/116(VentnActr01BusAdr_VentnActr01BusAdr_read)
  VentnActr01CoilSts_VentnActr01CoilSts/1 -> CCMVgaMotor_Runnable_sys/117(VentnActr01CoilSts_VentnActr01CoilSts_read)
  VentnActr01DrvDir_VentnActr01DrvDir/1 -> CCMVgaMotor_Runnable_sys/118(VentnActr01DrvDir_VentnActr01DrvDir_read)
  VentnActr01ElecErr_VentnActr01ElecErr/1 -> CCMVgaMotor_Runnable_sys/119(VentnActr01ElecErr_VentnActr01ElecErr_read)
  VentnActr01Mode_VentnActr01Mode/1 -> CCMVgaMotor_Runnable_sys/120(VentnActr01Mode_VentnActr01Mode_read)
  VentnActr01MovDir_VentnActr01MovDir/1 -> CCMVgaMotor_Runnable_sys/121(VentnActr01MovDir_VentnActr01MovDir_read)
  VentnActr01OvrTempErr_VentnActr01OvrTempErr/1 -> CCMVgaMotor_Runnable_sys/122(VentnActr01OvrTempErr_VentnActr01OvrTempErr_read)
  VentnActr01PsonSts_VentnActr01PsonSts/1 -> CCMVgaMotor_Runnable_sys/123(VentnActr01PsonSts_VentnActr01PsonSts_read)
  VentnActr01ResetSts_VentnActr01ResetSts/1 -> CCMVgaMotor_Runnable_sys/124(VentnActr01ResetSts_VentnActr01ResetSts_read)
  VentnActr01SpclFct_VentnActr01SpclFct/1 -> CCMVgaMotor_Runnable_sys/125(VentnActr01SpclFct_VentnActr01SpclFct_read)
  VentnActr01SpdSts_VentnActr01SpdSts/1 -> CCMVgaMotor_Runnable_sys/126(VentnActr01SpdSts_VentnActr01SpdSts_read)
  VentnActr01TrqSts_VentnActr01TrqSts/1 -> CCMVgaMotor_Runnable_sys/127(VentnActr01TrqSts_VentnActr01TrqSts_read)
  VentnActr01UrgcExist_VentnActr01UrgcExist/1 -> CCMVgaMotor_Runnable_sys/128(VentnActr01UrgcExist_VentnActr01UrgcExist_read)
  VentnActr01UrgcPson_VentnActr01UrgcPson/1 -> CCMVgaMotor_Runnable_sys/129(VentnActr01UrgcPson_VentnActr01UrgcPson_read)
  VentnActr01UrgcSts_VentnActr01UrgcSts/1 -> CCMVgaMotor_Runnable_sys/130(VentnActr01UrgcSts_VentnActr01UrgcSts_read)
  VentnActr01VoltErr_VentnActr01VoltErr/1 -> CCMVgaMotor_Runnable_sys/131(VentnActr01VoltErr_VentnActr01VoltErr_read)
  VentnActr02ActPson_VentnActr02ActPson/1 -> CCMVgaMotor_Runnable_sys/132(VentnActr02ActPson_VentnActr02ActPson_read)
  VentnActr02ActPson_VentnActr02ActPson_IsUpdated/1 -> CCMVgaMotor_Runnable_sys/133(VentnActr02ActPson_VentnActr02ActPson_IsUpdated_read)
  VentnActr02BlckInd_VentnActr02BlckInd/1 -> CCMVgaMotor_Runnable_sys/134(VentnActr02BlckInd_VentnActr02BlckInd_read)
  VentnActr02BlckSts_VentnActr02BlckSts/1 -> CCMVgaMotor_Runnable_sys/135(VentnActr02BlckSts_VentnActr02BlckSts_read)
  VentnActr02BusAdr_VentnActr02BusAdr/1 -> CCMVgaMotor_Runnable_sys/136(VentnActr02BusAdr_VentnActr02BusAdr_read)
  VentnActr02CoilSts_VentnActr02CoilSts/1 -> CCMVgaMotor_Runnable_sys/137(VentnActr02CoilSts_VentnActr02CoilSts_read)
  VentnActr02DrvDir_VentnActr02DrvDir/1 -> CCMVgaMotor_Runnable_sys/138(VentnActr02DrvDir_VentnActr02DrvDir_read)
  VentnActr02ElecErr_VentnActr02ElecErr/1 -> CCMVgaMotor_Runnable_sys/139(VentnActr02ElecErr_VentnActr02ElecErr_read)
  VentnActr02Mode_VentnActr02Mode/1 -> CCMVgaMotor_Runnable_sys/140(VentnActr02Mode_VentnActr02Mode_read)
  VentnActr02MovDir_VentnActr02MovDir/1 -> CCMVgaMotor_Runnable_sys/141(VentnActr02MovDir_VentnActr02MovDir_read)
  VentnActr02OvrTempErr_VentnActr02OvrTempErr/1 -> CCMVgaMotor_Runnable_sys/142(VentnActr02OvrTempErr_VentnActr02OvrTempErr_read)
  VentnActr02PsonSts_VentnActr02PsonSts/1 -> CCMVgaMotor_Runnable_sys/143(VentnActr02PsonSts_VentnActr02PsonSts_read)
  VentnActr02ResetSts_VentnActr02ResetSts/1 -> CCMVgaMotor_Runnable_sys/144(VentnActr02ResetSts_VentnActr02ResetSts_read)
  VentnActr02SpclFct_VentnActr02SpclFct/1 -> CCMVgaMotor_Runnable_sys/145(VentnActr02SpclFct_VentnActr02SpclFct_read)
  VentnActr02SpdSts_VentnActr02SpdSts/1 -> CCMVgaMotor_Runnable_sys/146(VentnActr02SpdSts_VentnActr02SpdSts_read)
  VentnActr02TrqSts_VentnActr02TrqSts/1 -> CCMVgaMotor_Runnable_sys/147(VentnActr02TrqSts_VentnActr02TrqSts_read)
  VentnActr02UrgcExist_VentnActr02UrgcExist/1 -> CCMVgaMotor_Runnable_sys/148(VentnActr02UrgcExist_VentnActr02UrgcExist_read)
  VentnActr02UrgcPson_VentnActr02UrgcPson/1 -> CCMVgaMotor_Runnable_sys/149(VentnActr02UrgcPson_VentnActr02UrgcPson_read)
  VentnActr02UrgcSts_VentnActr02UrgcSts/1 -> CCMVgaMotor_Runnable_sys/150(VentnActr02UrgcSts_VentnActr02UrgcSts_read)
  VentnActr02VoltErr_VentnActr02VoltErr/1 -> CCMVgaMotor_Runnable_sys/151(VentnActr02VoltErr_VentnActr02VoltErr_read)
  VentnActr03ActPson_VentnActr03ActPson/1 -> CCMVgaMotor_Runnable_sys/152(VentnActr03ActPson_VentnActr03ActPson_read)
  VentnActr03ActPson_VentnActr03ActPson_IsUpdated/1 -> CCMVgaMotor_Runnable_sys/153(VentnActr03ActPson_VentnActr03ActPson_IsUpdated_read)
  VentnActr03BlckInd_VentnActr03BlckInd/1 -> CCMVgaMotor_Runnable_sys/154(VentnActr03BlckInd_VentnActr03BlckInd_read)
  VentnActr03BlckSts_VentnActr03BlckSts/1 -> CCMVgaMotor_Runnable_sys/155(VentnActr03BlckSts_VentnActr03BlckSts_read)
  VentnActr03BusAdr_VentnActr03BusAdr/1 -> CCMVgaMotor_Runnable_sys/156(VentnActr03BusAdr_VentnActr03BusAdr_read)
  VentnActr03CoilSts_VentnActr03CoilSts/1 -> CCMVgaMotor_Runnable_sys/157(VentnActr03CoilSts_VentnActr03CoilSts_read)
  VentnActr03DrvDir_VentnActr03DrvDir/1 -> CCMVgaMotor_Runnable_sys/158(VentnActr03DrvDir_VentnActr03DrvDir_read)
  VentnActr03ElecErr_VentnActr03ElecErr/1 -> CCMVgaMotor_Runnable_sys/159(VentnActr03ElecErr_VentnActr03ElecErr_read)
  VentnActr03Mode_VentnActr03Mode/1 -> CCMVgaMotor_Runnable_sys/160(VentnActr03Mode_VentnActr03Mode_read)
  VentnActr03MovDir_VentnActr03MovDir/1 -> CCMVgaMotor_Runnable_sys/161(VentnActr03MovDir_VentnActr03MovDir_read)
  VentnActr03OvrTempErr_VentnActr03OvrTempErr/1 -> CCMVgaMotor_Runnable_sys/162(VentnActr03OvrTempErr_VentnActr03OvrTempErr_read)
  VentnActr03PsonSts_VentnActr03PsonSts/1 -> CCMVgaMotor_Runnable_sys/163(VentnActr03PsonSts_VentnActr03PsonSts_read)
  VentnActr03ResetSts_VentnActr03ResetSts/1 -> CCMVgaMotor_Runnable_sys/164(VentnActr03ResetSts_VentnActr03ResetSts_read)
  VentnActr03SpclFct_VentnActr03SpclFct/1 -> CCMVgaMotor_Runnable_sys/165(VentnActr03SpclFct_VentnActr03SpclFct_read)
  VentnActr03SpdSts_VentnActr03SpdSts/1 -> CCMVgaMotor_Runnable_sys/166(VentnActr03SpdSts_VentnActr03SpdSts_read)
  VentnActr03TrqSts_VentnActr03TrqSts/1 -> CCMVgaMotor_Runnable_sys/167(VentnActr03TrqSts_VentnActr03TrqSts_read)
  VentnActr03UrgcExist_VentnActr03UrgcExist/1 -> CCMVgaMotor_Runnable_sys/168(VentnActr03UrgcExist_VentnActr03UrgcExist_read)
  VentnActr03UrgcPson_VentnActr03UrgcPson/1 -> CCMVgaMotor_Runnable_sys/169(VentnActr03UrgcPson_VentnActr03UrgcPson_read)
  VentnActr03UrgcSts_VentnActr03UrgcSts/1 -> CCMVgaMotor_Runnable_sys/170(VentnActr03UrgcSts_VentnActr03UrgcSts_read)
  VentnActr03VoltErr_VentnActr03VoltErr/1 -> CCMVgaMotor_Runnable_sys/171(VentnActr03VoltErr_VentnActr03VoltErr_read)
  VentnActr04ActPson_VentnActr04ActPson/1 -> CCMVgaMotor_Runnable_sys/172(VentnActr04ActPson_VentnActr04ActPson_read)
  VentnActr04ActPson_VentnActr04ActPson_IsUpdated/1 -> CCMVgaMotor_Runnable_sys/173(VentnActr04ActPson_VentnActr04ActPson_IsUpdated_read)
  VentnActr04BlckInd_VentnActr04BlckInd/1 -> CCMVgaMotor_Runnable_sys/174(VentnActr04BlckInd_VentnActr04BlckInd_read)
  VentnActr04BlckSts_VentnActr04BlckSts/1 -> CCMVgaMotor_Runnable_sys/175(VentnActr04BlckSts_VentnActr04BlckSts_read)
  VentnActr04BusAdr_VentnActr04BusAdr/1 -> CCMVgaMotor_Runnable_sys/176(VentnActr04BusAdr_VentnActr04BusAdr_read)
  VentnActr04CoilSts_VentnActr04CoilSts/1 -> CCMVgaMotor_Runnable_sys/177(VentnActr04CoilSts_VentnActr04CoilSts_read)
  VentnActr04DrvDir_VentnActr04DrvDir/1 -> CCMVgaMotor_Runnable_sys/178(VentnActr04DrvDir_VentnActr04DrvDir_read)